simulink library "ee_lib_for_power_systems"  (R2025b)
settings: Solver=VariableStepAuto  StartTime=0.0  StopTime=10.0  RelTol=1e-3
undefined symbols (not defined in the file or in its folder): Alt_PolePair_Count, C3m, DiscIntNeverNeededParam, Enum1to12, L3m, Total_SM_Inertia, Ts
libraries: fl_lib x291, nesl_utility x219, ee_lib x139, custom_lib x12, $bdroot x10, ee_sl_lib x3, eeTransforms x2, eeGeneralControl x1, simulink x1
(part 9 of 11)

== system ee_lib_for_power_systems/Sense and Measure/voltage sensor ==
  PS-Simulink Converter1 : nesl_utility/PS-Simulink Converter  PhysicalDomain=network_engine_domain  SubClassName=ps_output  LeftPortType=input  RightPortType=output  PseudoPeriodic=off  Frequencies=[]  VectorFormat=inherit  Unit=inherit  AffineConversion=off
  Voltage Sensor : fl_lib/Electrical/Electrical Sensors/Voltage Sensor
  + : PMIOPort  Side=Left
  - : PMIOPort  Port=2  Side=Right
  v : Outport
  wires:
  PS-Simulink Converter1/1 -> v/1
  connections:
  Voltage Sensor/RConn2 -- -/RConn1
  PS-Simulink Converter1/LConn1 -- Voltage Sensor/RConn1
  Voltage Sensor/LConn1 -- +/RConn1

== system ee_lib_for_power_systems/Sources/Controlled Current Source ==
  CCS : Inport
  Controlled Current Source : fl_lib/Electrical/Electrical Sources/Controlled Current Source
  Simulink-PS Converter1 : nesl_utility/Simulink-PS Converter  PhysicalDomain=network_engine_domain  SubClassName=ps_input  LeftPortType=input  RightPortType=output  PseudoPeriodic=off  Frequencies=[]  NoiseDistribution=none  NoiseParameters=[]  Unit=1  AffineConversion=off  FilteringAndDerivatives=zero  SimscapeFilterOrder=1  InputFilterTimeConstant=0.001  UdotUserProvided=0
  + : PMIOPort  Side=Left
  - : PMIOPort  Port=2  Side=Right
  wires:
  CCS/1 -> Simulink-PS Converter1/1
  connections:
  Controlled Current Source/RConn2 -- -/RConn1
  Simulink-PS Converter1/RConn1 -- Controlled Current Source/RConn1
  Controlled Current Source/LConn1 -- +/RConn1

== system ee_lib_for_power_systems/Sources/Controlled Voltage Source ==
  CVS : Inport
  Controlled Voltage Source : fl_lib/Electrical/Electrical Sources/Controlled Voltage Source
  Simulink-PS Converter : nesl_utility/Simulink-PS Converter  PhysicalDomain=network_engine_domain  SubClassName=ps_input  LeftPortType=input  RightPortType=output  PseudoPeriodic=off  Frequencies=[]  NoiseDistribution=none  NoiseParameters=[]  Unit=1  AffineConversion=off  FilteringAndDerivatives=zero  SimscapeFilterOrder=1  InputFilterTimeConstant=0.001  UdotUserProvided=0
  + : PMIOPort  Side=Left
  - : PMIOPort  Port=2  Side=Right
  wires:
  CVS/1 -> Simulink-PS Converter/1
  connections:
  Simulink-PS Converter/RConn1 -- Controlled Voltage Source/RConn1
  Controlled Voltage Source/LConn1 -- +/RConn1
  Controlled Voltage Source/RConn2 -- -/RConn1

== system ee_lib_for_power_systems/Sources/Fuel Cell ==
  pfuel : Inport
  pair : Inport  Port=2
  qfuel : Inport  Port=3
  qair : Inport  Port=4
  Fuel Cell : ee_lib/Sources/Fuel Cell  model_fidelity=ee.enum.fuelcell.fidelity.detailed  Eoc=65 V  A=0.23 V  ri=0.05 Ohm  i0=80 A  i_lim=200 A  N_cell=65  number_unit=10  pH2_nom=1.5e5 Pa  pO2_nom=1.0e5 Pa  xH2=99  xO2=21  xW=1  dyn=ee.enum.include.no  Rd=0.005 Ohm  tau=10 s  Tnom=293.15 K  thermal_mass=30000 J/K  iFC_specify=off  iFC_priority=None  iFC=0 A  iFC_nominal_specify=off  iFC_nominal_value=1  iFC_nominal_unit=A  vFC_specify=off  vFC_priority=None  vFC=0 V  vFC_nominal_specify=off  vFC_nominal_value=1  vFC_nominal_unit=V  temperature_specify=off  temperature_priority=High  temperature=293 K  temperature_nominal_specify=off  temperature_nominal_value=1  temperature_nominal_unit=K
      settings: InternalSimscapePortConfiguration="{\"Bottom\":[],\"Left\":[{\"id\":\"H\",\"label\":\"H\",\"type\":\"foundation.thermal.thermal\"},{\"id\":\"pfuel\",\"label\":\"pfuel\",\"type\":\"input\"},{\"id\":\"pair\",\"label\":\"pair\",\"type\":\"input\"},{\"id\":\"qfuel\",\"label\":\"qfuel\",\"type\":\"input\"},{\"id\":\"qair\",\"label\":\"qair\",\"type\":\"input\"}],\"Right\":[{\"id\":\"p\",\"label\":\"+\",\"type\":\"foundation.electrical.electrical\"},{\"id\":\"n\",\"label\":\"-\",\"type\":\"foundation.electrical.electrical\"}],\"Top\":[]}"
  Simulink-PS Converter{,1..3} (4 alike) : nesl_utility/Simulink-PS Converter  PhysicalDomain=network_engine_domain  SubClassName=ps_input  LeftPortType=input  RightPortType=output  PseudoPeriodic=off  Frequencies=[]  NoiseDistribution=none  NoiseParameters=[]  Unit=1  AffineConversion=off  FilteringAndDerivatives=zero  SimscapeFilterOrder=1  InputFilterTimeConstant=0.001  UdotUserProvided=0
  Thermal Reference : fl_lib/Thermal/Thermal Elements/Thermal Reference
  + : PMIOPort  Side=Right
  - : PMIOPort  Port=2  Side=Right
  wires:
  pair/1 -> Simulink-PS Converter1/1
  qfuel/1 -> Simulink-PS Converter2/1
  pfuel/1 -> Simulink-PS Converter/1
  qair/1 -> Simulink-PS Converter3/1
  connections:
  Simulink-PS Converter/RConn1 -- Fuel Cell/LConn2
  Fuel Cell/RConn1 -- +/RConn1
  Simulink-PS Converter3/RConn1 -- Fuel Cell/LConn5
  Fuel Cell/LConn3 -- Simulink-PS Converter1/RConn1
  Simulink-PS Converter2/RConn1 -- Fuel Cell/LConn4
  Thermal Reference/LConn1 -- Fuel Cell/LConn1
  Fuel Cell/RConn2 -- -/RConn1

== system ee_lib_for_power_systems/Sources/Solar Array ==
  Ir : Inport
  Simulink-PS Converter : nesl_utility/Simulink-PS Converter  PhysicalDomain=network_engine_domain  SubClassName=ps_input  LeftPortType=input  RightPortType=output  PseudoPeriodic=off  Frequencies=[]  NoiseDistribution=none  NoiseParameters=[]  Unit=1  AffineConversion=off  FilteringAndDerivatives=zero  SimscapeFilterOrder=1  InputFilterTimeConstant=0.001  UdotUserProvided=0
  Solar Cell : ee_lib/Sources/Solar Cell  prm=ee.enum.sources.solar_cell_prm.datasheet  Isc=7.34 A  Voc=0.6 V  Is=1e-6 A  Is2=0 A  Iph=7.34 A  Ir0=1000 W/m^2  ec=1.5  N2=2  Rs=0 Ohm  Rp=inf Ohm  N_series=1  N_parallel=1  TIPH1=0 1/K  EG=1.11 eV  TXIS1=3  TXIS2=3  TRS1=0  TRP1=0  Tmeas=25 degC  TFIXED=25 degC  thermal_mass=100 J/K  initial_temperature=25 degC
  + : PMIOPort  Side=Right
  - : PMIOPort  Port=2  Side=Right
  wires:
  Ir/1 -> Simulink-PS Converter/1
  connections:
  Simulink-PS Converter/RConn1 -- Solar Cell/LConn1
  Solar Cell/LConn2 -- +/RConn1
  Solar Cell/RConn1 -- -/RConn1

== system ee_lib_for_power_systems/Transformations/Vectorized Park Transform ==
  abc : Inport
  theta : Inport  Port=2
  Constant : Constant  Value=-2*pi/3*ones(1,no_meas)  VectorParams1D=off  SampleTime=Ts
  Constant1 : Constant  Value=2*pi/3*ones(1,no_meas)  VectorParams1D=off  SampleTime=Ts
  Constant2 : Constant  Value=0.5*ones(1,no_meas)  VectorParams1D=off  SampleTime=Ts
  Constant3 : Constant  Value=0.5*ones(1,no_meas)  VectorParams1D=off  SampleTime=Ts
  Constant4 : Constant  Value=0.5*ones(1,no_meas)  VectorParams1D=off  SampleTime=Ts
  Gain : Gain  Gain=2/3
  Gain1 : Gain  Gain=2/3
  Gain2 : Gain  Gain=2/3
  Product : Product
  Product1 : Product
  Product2 : Product
  Sin : Trigonometry
  Sin1 : Trigonometry
  Sin2 : Trigonometry  Operator=cos
  Sin3 : Trigonometry  Operator=cos
  Sin6 : Trigonometry
  Sin7 : Trigonometry  Operator=cos
  Sum{1,5..7} (4 alike) : Sum  Inputs=++|
  Sum2 : Sum  Inputs=1  CollapseMode=Specified dimension  InputSameDT=on  SaturateOnIntegerOverflow=on  OutDataTypeStr=Inherit: Same as first input
  Sum3 : Sum  Inputs=1  CollapseMode=Specified dimension  InputSameDT=on  SaturateOnIntegerOverflow=on  OutDataTypeStr=Inherit: Same as first input
  Sum4 : Sum  Inputs=1  CollapseMode=Specified dimension  InputSameDT=on  SaturateOnIntegerOverflow=on  OutDataTypeStr=Inherit: Same as first input
  Vector Concatenate1 : Concatenate  NumInputs=3  Mode=Multidimensional array
  Vector Concatenate2 : Concatenate  NumInputs=3  Mode=Multidimensional array
  Vector Concatenate3 : Concatenate  NumInputs=3  Mode=Multidimensional array
  d : Outport  VectorParamsAs1DForOutWhenUnconnected=off
  q : Outport  Port=2  VectorParamsAs1DForOutWhenUnconnected=off
  0 : Outport  Port=3  VectorParamsAs1DForOutWhenUnconnected=off
  wires:
  theta/1 -> Sum7/1, Sin/1, Sum1/1, Sin2/1, Sum5/1, Sum6/1
  Sum7/1 -> Sin7/1
  Sum1/1 -> Sin3/1
  Constant1/1 -> Sum6/2, Sum7/2
  Constant/1 -> Sum5/2, Sum1/2
  Sum6/1 -> Sin6/1
  Sum5/1 -> Sin1/1
  abc/1 -> Product2/1, Product1/1, Product/1
  Sin/1 -> Vector Concatenate1/1
  Sin1/1 -> Vector Concatenate1/2
  Sin3/1 -> Vector Concatenate2/2
  Sin2/1 -> Vector Concatenate2/1
  Constant4/1 -> Vector Concatenate3/1
  Constant2/1 -> Vector Concatenate3/2
  Sin6/1 -> Vector Concatenate1/3
  Sin7/1 -> Vector Concatenate2/3
  Constant3/1 -> Vector Concatenate3/3
  Vector Concatenate3/1 -> Product2/2
  Vector Concatenate2/1 -> Product1/2
  Vector Concatenate1/1 -> Product/2
  Product/1 -> Sum2/1
  Product1/1 -> Sum3/1
  Product2/1 -> Sum4/1
  Sum3/1 -> Gain1/1
  Sum4/1 -> Gain2/1
  Sum2/1 -> Gain/1
  Gain2/1 -> 0/1
  Gain1/1 -> q/1
  Gain/1 -> d/1

== system ee_lib_for_power_systems/Transformations/Vectorized Inverse Park Transform ==
  dq0 : Inport
  theta : Inport  Port=2
  Constant : Constant  Value=-2*pi/3*ones(1,no_meas)  VectorParams1D=off  SampleTime=Ts
  Constant1 : Constant  Value=2*pi/3*ones(1,no_meas)  VectorParams1D=off  SampleTime=Ts
  Constant2 : Constant  Value=ones(1,no_meas)  VectorParams1D=off  SampleTime=Ts
  Constant3 : Constant  Value=ones(1,no_meas)  VectorParams1D=off  SampleTime=Ts
  Constant4 : Constant  Value=ones(1,no_meas)  VectorParams1D=off  SampleTime=Ts
  Product : Product
  Product1 : Product
  Product2 : Product
  Sin : Trigonometry
  Sin1 : Trigonometry  Operator=cos
  Sin2 : Trigonometry
  Sin3 : Trigonometry  Operator=cos
  Sin4 : Trigonometry
  Sin5 : Trigonometry  Operator=cos
  Sum : Sum  Inputs=++|
  Sum1 : Sum  Inputs=++|
  Sum2 : Sum  Inputs=1  CollapseMode=Specified dimension  InputSameDT=on  SaturateOnIntegerOverflow=on  OutDataTypeStr=Inherit: Same as first input
  Sum3 : Sum  Inputs=1  CollapseMode=Specified dimension  InputSameDT=on  SaturateOnIntegerOverflow=on  OutDataTypeStr=Inherit: Same as first input
  Sum4 : Sum  Inputs=1  CollapseMode=Specified dimension  InputSameDT=on  SaturateOnIntegerOverflow=on  OutDataTypeStr=Inherit: Same as first input
  Vector Concatenate1 : Concatenate  NumInputs=3  Mode=Multidimensional array
  Vector Concatenate2 : Concatenate  NumInputs=3  Mode=Multidimensional array
  Vector Concatenate3 : Concatenate  NumInputs=3  Mode=Multidimensional array
  a : Outport  VectorParamsAs1DForOutWhenUnconnected=off
  b : Outport  Port=2  VectorParamsAs1DForOutWhenUnconnected=off
  c : Outport  Port=3  VectorParamsAs1DForOutWhenUnconnected=off
  wires:
  Product2/1 -> Sum4/1
  Product1/1 -> Sum3/1
  Product/1 -> Sum2/1
  Vector Concatenate1/1 -> Product/2
  Vector Concatenate2/1 -> Product1/2
  Vector Concatenate3/1 -> Product2/2
  Constant3/1 -> Vector Concatenate3/3
  Constant4/1 -> Vector Concatenate2/3
  Constant2/1 -> Vector Concatenate1/3
  Sin5/1 -> Vector Concatenate3/2
  Sin4/1 -> Vector Concatenate3/1
  Sum1/1 -> Sin5/1, Sin4/1
  Constant1/1 -> Sum1/1
  Constant/1 -> Sum/1
  Sum/1 -> Sin2/1, Sin3/1
  Sin2/1 -> Vector Concatenate2/1
  Sin3/1 -> Vector Concatenate2/2
  Sin1/1 -> Vector Concatenate1/2
  Sin/1 -> Vector Concatenate1/1
  dq0/1 -> Product/1, Product1/1, Product2/1
  theta/1 -> Sum/2, Sin/1, Sum1/2, Sin1/1
  Sum2/1 -> a/1
  Sum3/1 -> b/1
  Sum4/1 -> c/1

== system ee_lib_for_power_systems/Utilities/Decoupling Bus ==
  V_in : Inport
  Controlled Voltage Source : fl_lib/Electrical/Electrical Sources/Controlled Voltage Source
  Controlled Voltage Source1 : fl_lib/Electrical/Electrical Sources/Controlled Voltage Source
  Controlled Voltage Source2 : fl_lib/Electrical/Electrical Sources/Controlled Voltage Source
  Demux : Demux  Outputs=3
  Electrical Reference2 : fl_lib/Electrical/Electrical Elements/Electrical Reference
  Mux1 : Mux  Inputs=3
  PS-Simulink Converter{,1,4..5,8..9} (6 alike) : nesl_utility/PS-Simulink Converter  PhysicalDomain=network_engine_domain  SubClassName=ps_output  LeftPortType=input  RightPortType=output  PseudoPeriodic=off  Frequencies=[]  VectorFormat=inherit  Unit=V*Hz*s  AffineConversion=off
  Phase Splitter : ee_lib/Connectors & References/Phase Splitter
  Resistor : fl_lib/Electrical/Electrical Elements/Resistor  R=Rcouple Ohm  i_specify=on  i_priority=Low  i=0 A  i_nominal_specify=off  i_nominal_value=1  i_nominal_unit=A  v_specify=on  v_priority=Low  v=0 V  v_nominal_specify=off  v_nominal_value=1  v_nominal_unit=V
  Resistor1 : fl_lib/Electrical/Electrical Elements/Resistor  R=Rcouple Ohm  i_specify=on  i_priority=Low  i=0 A  i_nominal_specify=off  i_nominal_value=1  i_nominal_unit=A  v_specify=on  v_priority=Low  v=0 V  v_nominal_specify=off  v_nominal_value=1  v_nominal_unit=V
  Resistor2 : fl_lib/Electrical/Electrical Elements/Resistor  R=Rcouple Ohm  i_specify=on  i_priority=Low  i=0 A  i_nominal_specify=off  i_nominal_value=1  i_nominal_unit=A  v_specify=on  v_priority=Low  v=0 V  v_nominal_specify=off  v_nominal_value=1  v_nominal_unit=V
  Simulink-PS Converter : nesl_utility/Simulink-PS Converter  PhysicalDomain=network_engine_domain  SubClassName=ps_input  LeftPortType=input  RightPortType=output  PseudoPeriodic=off  Frequencies=[]  NoiseDistribution=none  NoiseParameters=[]  Unit=1  AffineConversion=off  FilteringAndDerivatives=zero  SimscapeFilterOrder=1  InputFilterTimeConstant=0.001  UdotUserProvided=0
  Simulink-PS Converter1 : nesl_utility/Simulink-PS Converter  PhysicalDomain=network_engine_domain  SubClassName=ps_input  LeftPortType=input  RightPortType=output  PseudoPeriodic=off  Frequencies=[]  NoiseDistribution=none  NoiseParameters=[]  Unit=1  AffineConversion=off  FilteringAndDerivatives=zero  SimscapeFilterOrder=1  InputFilterTimeConstant=0.001  UdotUserProvided=0
  Simulink-PS Converter2 : nesl_utility/Simulink-PS Converter  PhysicalDomain=network_engine_domain  SubClassName=ps_input  LeftPortType=input  RightPortType=output  PseudoPeriodic=off  Frequencies=[]  NoiseDistribution=none  NoiseParameters=[]  Unit=1  AffineConversion=off  FilteringAndDerivatives=zero  SimscapeFilterOrder=1  InputFilterTimeConstant=0.001  UdotUserProvided=0
  Sum : Sum
  Sum1 : Sum
  Sum2 : Sum
  Voltage Sensor{,1..5} (6 alike) : fl_lib/Electrical/Electrical Sensors/Voltage Sensor
  ~ : PMIOPort  Side=Right
  V_out : Outport
  wires:
  PS-Simulink Converter5/1 -> Sum1/2
  PS-Simulink Converter1/1 -> Sum/2
  PS-Simulink Converter/1 -> Sum/1
  V_in/1 -> Demux/1
  Mux1/1 -> V_out/1
  Demux/3 -> Simulink-PS Converter1/1
  Demux/2 -> Simulink-PS Converter/1
  Demux/1 -> Simulink-PS Converter2/1
  Sum/1 -> Mux1/1
  PS-Simulink Converter4/1 -> Sum1/1
  Sum1/1 -> Mux1/2
  PS-Simulink Converter8/1 -> Sum2/1
  PS-Simulink Converter9/1 -> Sum2/2
  Sum2/1 -> Mux1/3
  connections:
  ~/RConn1 -- Phase Splitter/LConn1
  PS-Simulink Converter8/LConn1 -- Voltage Sensor5/RConn1
  PS-Simulink Converter9/LConn1 -- Voltage Sensor4/RConn1
  Voltage Sensor5/LConn1 -- Phase Splitter/RConn3 -- Resistor2/LConn1 -- Voltage Sensor4/LConn1
  Controlled Voltage Source2/LConn1 -- Voltage Sensor4/RConn2 -- Resistor2/RConn1
  Voltage Sensor1/RConn2 -- Voltage Sensor3/RConn2 -- Electrical Reference2/LConn1 -- Voltage Sensor5/RConn2 -- Controlled Voltage Source2/RConn2 -- Controlled Voltage Source1/RConn2 -- Controlled Voltage Source/RConn2
  PS-Simulink Converter5/LConn1 -- Voltage Sensor2/RConn1
  PS-Simulink Converter4/LConn1 -- Voltage Sensor3/RConn1
  Phase Splitter/RConn2 -- Voltage Sensor2/LConn1 -- Resistor1/LConn1 -- Voltage Sensor3/LConn1
  Controlled Voltage Source1/LConn1 -- Resistor1/RConn1 -- Voltage Sensor2/RConn2
  PS-Simulink Converter/LConn1 -- Voltage Sensor1/RConn1
  PS-Simulink Converter1/LConn1 -- Voltage Sensor/RConn1
  Voltage Sensor/LConn1 -- Voltage Sensor1/LConn1 -- Phase Splitter/RConn1 -- Resistor/LConn1
  Controlled Voltage Source/LConn1 -- Voltage Sensor/RConn2 -- Resistor/RConn1
  Simulink-PS Converter2/RConn1 -- Controlled Voltage Source/RConn1
  Simulink-PS Converter/RConn1 -- Controlled Voltage Source1/RConn1
  Simulink-PS Converter1/RConn1 -- Controlled Voltage Source2/RConn1

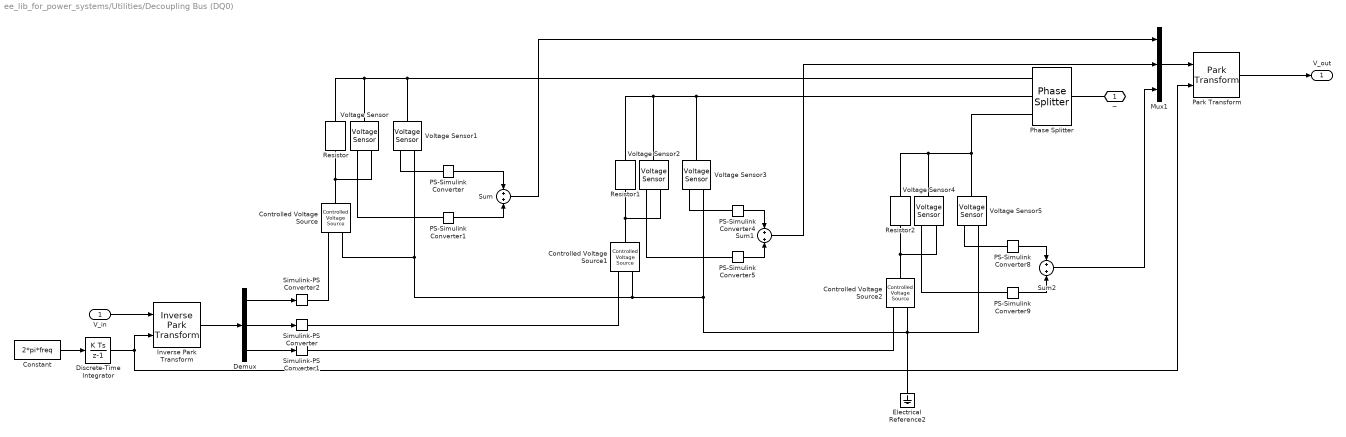
== system ee_lib_for_power_systems/Utilities/Decoupling Bus (DQ0) ==
  V_in : Inport
  Constant : Constant  Value=2*pi*freq  SampleTime=-1
  Controlled Voltage Source : fl_lib/Electrical/Electrical Sources/Controlled Voltage Source
  Controlled Voltage Source1 : fl_lib/Electrical/Electrical Sources/Controlled Voltage Source
  Controlled Voltage Source2 : fl_lib/Electrical/Electrical Sources/Controlled Voltage Source
  Demux : Demux  Outputs=3
  Discrete-Time Integrator : DiscreteIntegrator  SampleTime=-1  ICPrevOutput=DiscIntNeverNeededParam  ICPrevScaledInput=DiscIntNeverNeededParam
  Electrical Reference2 : fl_lib/Electrical/Electrical Elements/Electrical Reference
  Inverse Park Transform : eeTransforms/Inverse Park Transform  AxisAlignment=Q-axis  PowerInvariant=off
      settings: LibrarySourceBlock=|
      | ee_sl_lib/Mathematical
      | Transforms/Inverse
      | Park Transform
  Mux1 : Mux  Inputs=3
  PS-Simulink Converter{,1,4..5,8..9} (6 alike) : nesl_utility/PS-Simulink Converter  PhysicalDomain=network_engine_domain  SubClassName=ps_output  LeftPortType=input  RightPortType=output  PseudoPeriodic=off  Frequencies=[]  VectorFormat=inherit  Unit=V*Hz*s  AffineConversion=off
  Park Transform : eeTransforms/Park Transform  AxisAlignment=Q-axis  PowerInvariant=off
      settings: LibrarySourceBlock=|
      | ee_sl_lib/Mathematical
      | Transforms/Park Transform
  Phase Splitter : ee_lib/Connectors & References/Phase Splitter
  Resistor : fl_lib/Electrical/Electrical Elements/Resistor  R=Rcouple Ohm  i_specify=on  i_priority=Low  i=0 A  i_nominal_specify=off  i_nominal_value=1  i_nominal_unit=A  v_specify=on  v_priority=Low  v=0 V  v_nominal_specify=off  v_nominal_value=1  v_nominal_unit=V
  Resistor1 : fl_lib/Electrical/Electrical Elements/Resistor  R=Rcouple Ohm  i_specify=on  i_priority=Low  i=0 A  i_nominal_specify=off  i_nominal_value=1  i_nominal_unit=A  v_specify=on  v_priority=Low  v=0 V  v_nominal_specify=off  v_nominal_value=1  v_nominal_unit=V
  Resistor2 : fl_lib/Electrical/Electrical Elements/Resistor  R=Rcouple Ohm  i_specify=on  i_priority=Low  i=0 A  i_nominal_specify=off  i_nominal_value=1  i_nominal_unit=A  v_specify=on  v_priority=Low  v=0 V  v_nominal_specify=off  v_nominal_value=1  v_nominal_unit=V
  Simulink-PS Converter : nesl_utility/Simulink-PS Converter  PhysicalDomain=network_engine_domain  SubClassName=ps_input  LeftPortType=input  RightPortType=output  PseudoPeriodic=off  Frequencies=[]  NoiseDistribution=none  NoiseParameters=[]  Unit=1  AffineConversion=off  FilteringAndDerivatives=zero  SimscapeFilterOrder=1  InputFilterTimeConstant=0.001  UdotUserProvided=0
  Simulink-PS Converter1 : nesl_utility/Simulink-PS Converter  PhysicalDomain=network_engine_domain  SubClassName=ps_input  LeftPortType=input  RightPortType=output  PseudoPeriodic=off  Frequencies=[]  NoiseDistribution=none  NoiseParameters=[]  Unit=1  AffineConversion=off  FilteringAndDerivatives=zero  SimscapeFilterOrder=1  InputFilterTimeConstant=0.001  UdotUserProvided=0
  Simulink-PS Converter2 : nesl_utility/Simulink-PS Converter  PhysicalDomain=network_engine_domain  SubClassName=ps_input  LeftPortType=input  RightPortType=output  PseudoPeriodic=off  Frequencies=[]  NoiseDistribution=none  NoiseParameters=[]  Unit=1  AffineConversion=off  FilteringAndDerivatives=zero  SimscapeFilterOrder=1  InputFilterTimeConstant=0.001  UdotUserProvided=0
  Sum : Sum
  Sum1 : Sum
  Sum2 : Sum
  Voltage Sensor{,1..5} (6 alike) : fl_lib/Electrical/Electrical Sensors/Voltage Sensor
  ~ : PMIOPort  Side=Right
  V_out : Outport
  wires:
  Sum2/1 -> Mux1/3
  PS-Simulink Converter9/1 -> Sum2/2
  PS-Simulink Converter8/1 -> Sum2/1
  Sum1/1 -> Mux1/2
  PS-Simulink Converter4/1 -> Sum1/1
  Sum/1 -> Mux1/1
  Demux/1 -> Simulink-PS Converter2/1
  Demux/2 -> Simulink-PS Converter/1
  Demux/3 -> Simulink-PS Converter1/1
  PS-Simulink Converter/1 -> Sum/1
  PS-Simulink Converter1/1 -> Sum/2
  PS-Simulink Converter5/1 -> Sum1/2
  Inverse Park Transform/1 -> Demux/1
  V_in/1 -> Inverse Park Transform/1
  Discrete-Time Integrator/1 -> Inverse Park Transform/2, Park Transform/2
  Constant/1 -> Discrete-Time Integrator/1
  Mux1/1 -> Park Transform/1
  Park Transform/1 -> V_out/1
  connections:
  Simulink-PS Converter1/RConn1 -- Controlled Voltage Source2/RConn1
  Simulink-PS Converter/RConn1 -- Controlled Voltage Source1/RConn1
  Simulink-PS Converter2/RConn1 -- Controlled Voltage Source/RConn1
  Resistor/RConn1 -- Voltage Sensor/RConn2 -- Controlled Voltage Source/LConn1
  Resistor/LConn1 -- Phase Splitter/RConn1 -- Voltage Sensor1/LConn1 -- Voltage Sensor/LConn1
  PS-Simulink Converter1/LConn1 -- Voltage Sensor/RConn1
  PS-Simulink Converter/LConn1 -- Voltage Sensor1/RConn1
  Voltage Sensor2/RConn2 -- Resistor1/RConn1 -- Controlled Voltage Source1/LConn1
  Voltage Sensor3/LConn1 -- Voltage Sensor2/LConn1 -- Resistor1/LConn1 -- Phase Splitter/RConn2
  PS-Simulink Converter4/LConn1 -- Voltage Sensor3/RConn1
  PS-Simulink Converter5/LConn1 -- Voltage Sensor2/RConn1
  Controlled Voltage Source/RConn2 -- Controlled Voltage Source1/RConn2 -- Electrical Reference2/LConn1 -- Controlled Voltage Source2/RConn2 -- Voltage Sensor5/RConn2 -- Voltage Sensor3/RConn2 -- Voltage Sensor1/RConn2
  Resistor2/RConn1 -- Voltage Sensor4/RConn2 -- Controlled Voltage Source2/LConn1
  Voltage Sensor4/LConn1 -- Resistor2/LConn1 -- Phase Splitter/RConn3 -- Voltage Sensor5/LConn1
  PS-Simulink Converter9/LConn1 -- Voltage Sensor4/RConn1
  PS-Simulink Converter8/LConn1 -- Voltage Sensor5/RConn1
  ~/RConn1 -- Phase Splitter/LConn1

== system ee_lib_for_power_systems/Utilities/VF Replay PU (Three-Phase) ==
  V (pu) : Inport
  Freq (pu) : Inport  Port=2
  Constant : Constant  Value=[0 -1 1]*2*pi/3  SampleTime=Ts
  Controlled Voltage Source (Three-Phase) : ee_lib/Sources/Controlled Voltage Source (Three-Phase)  input_option=ee.enum.inputType.instantaneous  FRated=60 Hz
      settings: InternalSimscapePortConfiguration="{\"Bottom\":[],\"Left\":[{\"id\":\"S\",\"label\":\"S\",\"type\":\"input\"},{\"id\":\"n\",\"label\":\"n\",\"type\":\"foundation.electrical.electrical\"}],\"Right\":[{\"id\":\"N\",\"label\":\"~\",\"type\":\"foundation.electrical.three_phase\"}],\"Top\":[]}"
  Discrete-Time Integrator : DiscreteIntegrator  SampleTime=Ts  ICPrevOutput=DiscIntNeverNeededParam  ICPrevScaledInput=DiscIntNeverNeededParam
  Electrical Reference : fl_lib/Electrical/Electrical Elements/Electrical Reference
  Gain : Gain  Gain=2*pi*Fnom
  Gain1 : Gain  Gain=Vnom*sqrt(2/3)
  Product : Product
  Simulink-PS Converter : nesl_utility/Simulink-PS Converter  PhysicalDomain=network_engine_domain  SubClassName=ps_input  LeftPortType=input  RightPortType=output  PseudoPeriodic=off  Frequencies=[]  NoiseDistribution=none  NoiseParameters=[]  Unit=1  AffineConversion=off  FilteringAndDerivatives=zero  SimscapeFilterOrder=1  InputFilterTimeConstant=0.001  UdotUserProvided=0
  Sin : Trigonometry
  Sum : Sum  Inputs=|++
  ~ : PMIOPort  Side=Right
  wires:
  Gain1/1 -> Product/1
  V (pu)/1 -> Gain1/1
  Gain/1 -> Discrete-Time Integrator/1
  Discrete-Time Integrator/1 -> Sum/1
  Constant/1 -> Sum/2
  Sin/1 -> Product/2
  Sum/1 -> Sin/1
  Freq (pu)/1 -> Gain/1
  Product/1 -> Simulink-PS Converter/1
  connections:
  Controlled Voltage Source (Three-Phase)/RConn1 -- ~/RConn1
  Electrical Reference/LConn1 -- Controlled Voltage Source (Three-Phase)/LConn2
  Simulink-PS Converter/RConn1 -- Controlled Voltage Source (Three-Phase)/LConn1

== system ee_lib_for_power_systems/Utilities/VF Replay SI (Three-Phase) ==
  V (Vrms) : Inport
  Freq (Hz) : Inport  Port=2
  Constant : Constant  Value=[0 -1 1]*2*pi/3  SampleTime=Ts
  Controlled Voltage Source (Three-Phase) : ee_lib/Sources/Controlled Voltage Source (Three-Phase)  input_option=ee.enum.inputType.instantaneous  FRated=60 Hz
      settings: InternalSimscapePortConfiguration="{\"Bottom\":[],\"Left\":[{\"id\":\"S\",\"label\":\"S\",\"type\":\"input\"},{\"id\":\"n\",\"label\":\"n\",\"type\":\"foundation.electrical.electrical\"}],\"Right\":[{\"id\":\"N\",\"label\":\"~\",\"type\":\"foundation.electrical.three_phase\"}],\"Top\":[]}"
  Discrete-Time Integrator : DiscreteIntegrator  SampleTime=Ts  ICPrevOutput=DiscIntNeverNeededParam  ICPrevScaledInput=DiscIntNeverNeededParam
  Electrical Reference : fl_lib/Electrical/Electrical Elements/Electrical Reference
  Gain : Gain  Gain=2*pi
  Gain1 : Gain  Gain=sqrt(2/3)
  Product : Product
  Simulink-PS Converter : nesl_utility/Simulink-PS Converter  PhysicalDomain=network_engine_domain  SubClassName=ps_input  LeftPortType=input  RightPortType=output  PseudoPeriodic=off  Frequencies=[]  NoiseDistribution=none  NoiseParameters=[]  Unit=1  AffineConversion=off  FilteringAndDerivatives=zero  SimscapeFilterOrder=1  InputFilterTimeConstant=0.001  UdotUserProvided=0
  Sin : Trigonometry
  Sum : Sum  Inputs=|++
  ~ : PMIOPort  Side=Right
  wires:
  Product/1 -> Simulink-PS Converter/1
  Freq (Hz)/1 -> Gain/1
  Sum/1 -> Sin/1
  Sin/1 -> Product/2
  Constant/1 -> Sum/2
  Discrete-Time Integrator/1 -> Sum/1
  Gain/1 -> Discrete-Time Integrator/1
  V (Vrms)/1 -> Gain1/1
  Gain1/1 -> Product/1
  connections:
  Simulink-PS Converter/RConn1 -- Controlled Voltage Source (Three-Phase)/LConn1
  Electrical Reference/LConn1 -- Controlled Voltage Source (Three-Phase)/LConn2
  Controlled Voltage Source (Three-Phase)/RConn1 -- ~/RConn1

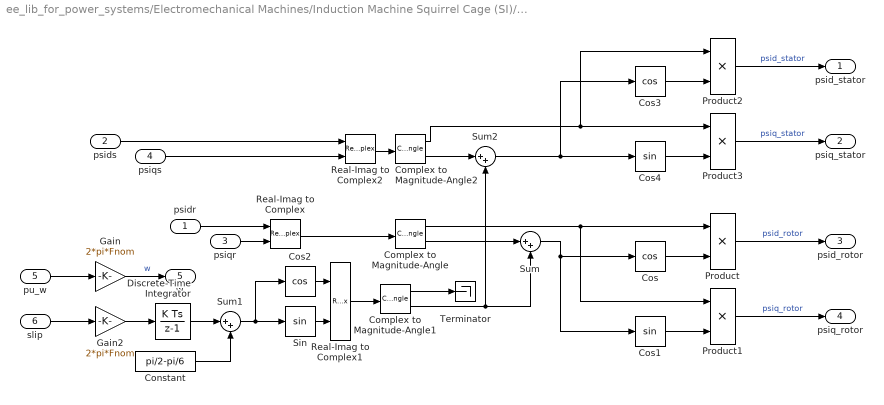
== system ee_lib_for_power_systems/Electromechanical Machines/Induction Machine Squirrel Cage (SI)/Subsystem ==
  psidr : Inport
  psids : Inport  Port=2
  psiqr : Inport  Port=3
  psiqs : Inport  Port=4
  pu_w : Inport  Port=5
  slip : Inport  Port=6
  Complex to Magnitude-Angle : ComplexToMagnitudeAngle
  Complex to Magnitude-Angle1 : ComplexToMagnitudeAngle
  Complex to Magnitude-Angle2 : ComplexToMagnitudeAngle
  Constant : Constant  Value=pi/2-pi/6  SampleTime=-1
  Cos : Trigonometry  Operator=cos
  Cos1 : Trigonometry
  Cos2 : Trigonometry  Operator=cos
  Cos3 : Trigonometry  Operator=cos
  Cos4 : Trigonometry
  Discrete-Time Integrator : DiscreteIntegrator  SampleTime=-1  ICPrevOutput=DiscIntNeverNeededParam  ICPrevScaledInput=DiscIntNeverNeededParam
  Gain : Gain  Gain=2*pi*Fnom
  Gain2 : Gain  Gain=2*pi*Fnom
  Product{,1..3} (4 alike) : Product
  Real-Imag to Complex : RealImagToComplex
  Real-Imag to Complex1 : RealImagToComplex
  Real-Imag to Complex2 : RealImagToComplex
  Sin : Trigonometry
  Sum : Sum  Inputs=|++
  Sum1 : Sum  Inputs=|++
  Sum2 : Sum  Inputs=|++
  Terminator : Terminator
  psid_stator : Outport
  psiq_stator : Outport  Port=2
  psid_rotor : Outport  Port=3
  psiq_rotor : Outport  Port=4
  w : Outport  Port=5
  wires:
  Cos/1 -> Product/2
  Gain2/1 -> Discrete-Time Integrator/1
  Sum2/1 -> Cos3/1, Cos4/1
  Constant/1 -> Sum1/2
  Sin/1 -> Real-Imag to Complex1/2
  Sum1/1 -> Cos2/1, Sin/1
  Complex to Magnitude-Angle2/1 -> Product2/1, Product3/1
  Complex to Magnitude-Angle/2 -> Sum/1
  Real-Imag to Complex1/1 -> Complex to Magnitude-Angle1/1
  Cos1/1 -> Product1/2
  Complex to Magnitude-Angle/1 -> Product1/1, Product/1
  psidr/1 -> Real-Imag to Complex/1
  Real-Imag to Complex/1 -> Complex to Magnitude-Angle/1
  Cos2/1 -> Real-Imag to Complex1/1
  Product/1 -> psid_rotor/1  "psid_rotor"
  Cos3/1 -> Product2/2
  Sum/1 -> Cos1/1, Cos/1
  Discrete-Time Integrator/1 -> Sum1/1
  Complex to Magnitude-Angle1/2 -> Sum2/2, Sum/2
  Cos4/1 -> Product3/2
  Product3/1 -> psiq_stator/1  "psiq_stator"
  psiqr/1 -> Real-Imag to Complex/2
  Real-Imag to Complex2/1 -> Complex to Magnitude-Angle2/1
  psiqs/1 -> Real-Imag to Complex2/2
  Complex to Magnitude-Angle2/2 -> Sum2/1
  pu_w/1 -> Gain/1
  Product2/1 -> psid_stator/1  "psid_stator"
  Product1/1 -> psiq_rotor/1  "psiq_rotor"
  psids/1 -> Real-Imag to Complex2/1
  Gain/1 -> w/1  "w"
  Complex to Magnitude-Angle1/1 -> Terminator/1
  slip/1 -> Gain2/1

== system ee_lib_for_power_systems/Electromechanical Machines/Round Rotor Standard (PU)/Meas ==
  Bus Creator : BusCreator  Inputs=4
  Mux : Mux  Inputs=2
  PS-Simulink Converter{,1..4} (5 alike) : nesl_utility/PS-Simulink Converter  PhysicalDomain=network_engine_domain  SubClassName=ps_output  LeftPortType=input  RightPortType=output  PseudoPeriodic=off  Frequencies=[]  VectorFormat=inherit  Unit=inherit  AffineConversion=off
  Sqrt : Sqrt
  Square : Math  Operator=square
  Sum : Sum  Inputs=+
  Synchronous Machine Measurement : ee_lib/Electromechanical/Synchronous/Synchronous Machine Measurement  output_idx=4
      settings: InternalSimscapePortConfiguration="{\"Bottom\":[],\"Left\":[{\"id\":\"pu_input\",\"label\":\"pu\",\"type\":\"input\"}],\"Right\":[{\"id\":\"pu_output\",\"label\":\"o\",\"type\":\"output\"}],\"Top\":[]}"
  Synchronous Machine Measurement1 : ee_lib/Electromechanical/Synchronous/Synchronous Machine Measurement  output_idx=5
      settings: InternalSimscapePortConfiguration="{\"Bottom\":[],\"Left\":[{\"id\":\"pu_input\",\"label\":\"pu\",\"type\":\"input\"}],\"Right\":[{\"id\":\"pu_output\",\"label\":\"o\",\"type\":\"output\"}],\"Top\":[]}"
  Synchronous Machine Measurement2 : ee_lib/Electromechanical/Synchronous/Synchronous Machine Measurement  output_idx=6
      settings: InternalSimscapePortConfiguration="{\"Bottom\":[],\"Left\":[{\"id\":\"pu_input\",\"label\":\"pu\",\"type\":\"input\"}],\"Right\":[{\"id\":\"pu_output\",\"label\":\"o\",\"type\":\"output\"}],\"Top\":[]}"
  Synchronous Machine Measurement3 : ee_lib/Electromechanical/Synchronous/Synchronous Machine Measurement  output_idx=12
      settings: InternalSimscapePortConfiguration="{\"Bottom\":[],\"Left\":[{\"id\":\"pu_input\",\"label\":\"pu\",\"type\":\"input\"}],\"Right\":[{\"id\":\"pu_output\",\"label\":\"o\",\"type\":\"output\"}],\"Top\":[]}"
  Synchronous Machine Measurement4 : ee_lib/Electromechanical/Synchronous/Synchronous Machine Measurement  output_idx=13
      settings: InternalSimscapePortConfiguration="{\"Bottom\":[],\"Left\":[{\"id\":\"pu_input\",\"label\":\"pu\",\"type\":\"input\"}],\"Right\":[{\"id\":\"pu_output\",\"label\":\"o\",\"type\":\"output\"}],\"Top\":[]}"
  m_PS : PMIOPort  Side=Left
  m : Outport
  wires:
  PS-Simulink Converter/1 -> Bus Creator/1  "w (pu)"
  Bus Creator/1 -> m/1
  PS-Simulink Converter1/1 -> Mux/1
  PS-Simulink Converter2/1 -> Mux/2
  Mux/1 -> Square/1
  Square/1 -> Sum/1
  Sum/1 -> Sqrt/1
  PS-Simulink Converter4/1 -> Bus Creator/4  "Q (pu)"
  Sqrt/1 -> Bus Creator/2  "Vt (pu)"
  PS-Simulink Converter3/1 -> Bus Creator/3  "P (pu)"
  connections:
  PS-Simulink Converter2/LConn1 -- Synchronous Machine Measurement2/RConn1
  PS-Simulink Converter/LConn1 -- Synchronous Machine Measurement/RConn1
  Synchronous Machine Measurement4/LConn1 -- Synchronous Machine Measurement3/LConn1 -- m_PS/RConn1 -- Synchronous Machine Measurement2/LConn1 -- Synchronous Machine Measurement1/LConn1 -- Synchronous Machine Measurement/LConn1
  PS-Simulink Converter4/LConn1 -- Synchronous Machine Measurement4/RConn1
  PS-Simulink Converter3/LConn1 -- Synchronous Machine Measurement3/RConn1
  PS-Simulink Converter1/LConn1 -- Synchronous Machine Measurement1/RConn1

== system ee_lib_for_power_systems/Electromechanical Machines/Salient Pole Fundamental (SI)/Meas ==
  Bus Creator : BusCreator  Inputs=7
  Gain : Gain  Gain=2*pi*Fnom/p
  Gain1 : Gain  Gain=Pnom/Vnom*sqrt(2/3)
  Gain2 : Gain  Gain=Vnom*sqrt(2/3)
  Gain3 : Gain  Gain=Vnom*sqrt(2/3)
  Gain4 : Gain  Gain=Pnom
  Gain5 : Gain  Gain=Pnom
  Mux : Mux  Inputs=2
  PS-Simulink Converter{,1..5} (6 alike) : nesl_utility/PS-Simulink Converter  PhysicalDomain=network_engine_domain  SubClassName=ps_output  LeftPortType=input  RightPortType=output  PseudoPeriodic=off  Frequencies=[]  VectorFormat=inherit  Unit=inherit  AffineConversion=off
  Sqrt : Sqrt
  Square : Math  Operator=square
  Sum : Sum  Inputs=+
  Synchronous Machine Measurement : ee_lib/Electromechanical/Synchronous/Synchronous Machine Measurement  output_idx=4
      settings: InternalSimscapePortConfiguration="{\"Bottom\":[],\"Left\":[{\"id\":\"pu_input\",\"label\":\"pu\",\"type\":\"input\"}],\"Right\":[{\"id\":\"pu_output\",\"label\":\"o\",\"type\":\"output\"}],\"Top\":[]}"
  Synchronous Machine Measurement1 : ee_lib/Electromechanical/Synchronous/Synchronous Machine Measurement  output_idx=5
      settings: InternalSimscapePortConfiguration="{\"Bottom\":[],\"Left\":[{\"id\":\"pu_input\",\"label\":\"pu\",\"type\":\"input\"}],\"Right\":[{\"id\":\"pu_output\",\"label\":\"o\",\"type\":\"output\"}],\"Top\":[]}"
  Synchronous Machine Measurement2 : ee_lib/Electromechanical/Synchronous/Synchronous Machine Measurement  output_idx=6
      settings: InternalSimscapePortConfiguration="{\"Bottom\":[],\"Left\":[{\"id\":\"pu_input\",\"label\":\"pu\",\"type\":\"input\"}],\"Right\":[{\"id\":\"pu_output\",\"label\":\"o\",\"type\":\"output\"}],\"Top\":[]}"
  Synchronous Machine Measurement3 : ee_lib/Electromechanical/Synchronous/Synchronous Machine Measurement  output_idx=12
      settings: InternalSimscapePortConfiguration="{\"Bottom\":[],\"Left\":[{\"id\":\"pu_input\",\"label\":\"pu\",\"type\":\"input\"}],\"Right\":[{\"id\":\"pu_output\",\"label\":\"o\",\"type\":\"output\"}],\"Top\":[]}"
  Synchronous Machine Measurement4 : ee_lib/Electromechanical/Synchronous/Synchronous Machine Measurement  output_idx=13
      settings: InternalSimscapePortConfiguration="{\"Bottom\":[],\"Left\":[{\"id\":\"pu_input\",\"label\":\"pu\",\"type\":\"input\"}],\"Right\":[{\"id\":\"pu_output\",\"label\":\"o\",\"type\":\"output\"}],\"Top\":[]}"
  Synchronous Machine Measurement5 : ee_lib/Electromechanical/Synchronous/Synchronous Machine Measurement  output_idx=2
      settings: InternalSimscapePortConfiguration="{\"Bottom\":[],\"Left\":[{\"id\":\"pu_input\",\"label\":\"pu\",\"type\":\"input\"}],\"Right\":[{\"id\":\"pu_output\",\"label\":\"o\",\"type\":\"output\"}],\"Top\":[]}"
  m_PS : PMIOPort  Side=Left
  m : Outport
  wires:
  Sqrt/1 -> Bus Creator/5  "Vt (V)"
  Sum/1 -> Sqrt/1
  Square/1 -> Sum/1
  Mux/1 -> Square/1
  PS-Simulink Converter2/1 -> Gain3/1
  PS-Simulink Converter1/1 -> Gain2/1
  Bus Creator/1 -> m/1
  PS-Simulink Converter/1 -> Gain/1  "w (pu)"
  PS-Simulink Converter5/1 -> Gain1/1  "Ifd (pu)"
  PS-Simulink Converter3/1 -> Gain4/1  "P (pu)"
  PS-Simulink Converter4/1 -> Gain5/1  "Q (pu)"
  Gain/1 -> Bus Creator/1  "w (rad/s)"
  Gain1/1 -> Bus Creator/2  "Ifd (A)"
  Gain2/1 -> Bus Creator/3, Mux/1  "Vd (V)"
  Gain3/1 -> Bus Creator/4, Mux/2  "Vq (V)"
  Gain4/1 -> Bus Creator/6  "P (W)"
  Gain5/1 -> Bus Creator/7  "Q (VAr)"
  connections:
  PS-Simulink Converter1/LConn1 -- Synchronous Machine Measurement1/RConn1
  PS-Simulink Converter3/LConn1 -- Synchronous Machine Measurement3/RConn1
  PS-Simulink Converter4/LConn1 -- Synchronous Machine Measurement4/RConn1
  Synchronous Machine Measurement5/LConn1 -- Synchronous Machine Measurement1/LConn1 -- Synchronous Machine Measurement2/LConn1 -- m_PS/RConn1 -- Synchronous Machine Measurement3/LConn1 -- Synchronous Machine Measurement4/LConn1 -- Synchronous Machine Measurement/LConn1
  PS-Simulink Converter/LConn1 -- Synchronous Machine Measurement/RConn1
  PS-Simulink Converter2/LConn1 -- Synchronous Machine Measurement2/RConn1
  PS-Simulink Converter5/LConn1 -- Synchronous Machine Measurement5/RConn1

== system ee_lib_for_power_systems/Electromechanical Machines/Salient Pole Standard (PU)/Meas ==
  Bus Creator : BusCreator  Inputs=4
  Mux : Mux  Inputs=2
  PS-Simulink Converter{,1..4} (5 alike) : nesl_utility/PS-Simulink Converter  PhysicalDomain=network_engine_domain  SubClassName=ps_output  LeftPortType=input  RightPortType=output  PseudoPeriodic=off  Frequencies=[]  VectorFormat=inherit  Unit=inherit  AffineConversion=off
  Sqrt : Sqrt
  Square : Math  Operator=square
  Sum : Sum  Inputs=+
  Synchronous Machine Measurement : ee_lib/Electromechanical/Synchronous/Synchronous Machine Measurement  output_idx=4
      settings: InternalSimscapePortConfiguration="{\"Bottom\":[],\"Left\":[{\"id\":\"pu_input\",\"label\":\"pu\",\"type\":\"input\"}],\"Right\":[{\"id\":\"pu_output\",\"label\":\"o\",\"type\":\"output\"}],\"Top\":[]}"
  Synchronous Machine Measurement1 : ee_lib/Electromechanical/Synchronous/Synchronous Machine Measurement  output_idx=5
      settings: InternalSimscapePortConfiguration="{\"Bottom\":[],\"Left\":[{\"id\":\"pu_input\",\"label\":\"pu\",\"type\":\"input\"}],\"Right\":[{\"id\":\"pu_output\",\"label\":\"o\",\"type\":\"output\"}],\"Top\":[]}"
  Synchronous Machine Measurement2 : ee_lib/Electromechanical/Synchronous/Synchronous Machine Measurement  output_idx=6
      settings: InternalSimscapePortConfiguration="{\"Bottom\":[],\"Left\":[{\"id\":\"pu_input\",\"label\":\"pu\",\"type\":\"input\"}],\"Right\":[{\"id\":\"pu_output\",\"label\":\"o\",\"type\":\"output\"}],\"Top\":[]}"
  Synchronous Machine Measurement3 : ee_lib/Electromechanical/Synchronous/Synchronous Machine Measurement  output_idx=12
      settings: InternalSimscapePortConfiguration="{\"Bottom\":[],\"Left\":[{\"id\":\"pu_input\",\"label\":\"pu\",\"type\":\"input\"}],\"Right\":[{\"id\":\"pu_output\",\"label\":\"o\",\"type\":\"output\"}],\"Top\":[]}"
  Synchronous Machine Measurement4 : ee_lib/Electromechanical/Synchronous/Synchronous Machine Measurement  output_idx=13
      settings: InternalSimscapePortConfiguration="{\"Bottom\":[],\"Left\":[{\"id\":\"pu_input\",\"label\":\"pu\",\"type\":\"input\"}],\"Right\":[{\"id\":\"pu_output\",\"label\":\"o\",\"type\":\"output\"}],\"Top\":[]}"
  m_PS : PMIOPort  Side=Left
  m : Outport
  wires:
  PS-Simulink Converter3/1 -> Bus Creator/3  "P (pu)"
  Sqrt/1 -> Bus Creator/2  "Vt (pu)"
  PS-Simulink Converter4/1 -> Bus Creator/4  "Q (pu)"
  Sum/1 -> Sqrt/1
  Square/1 -> Sum/1
  Mux/1 -> Square/1
  PS-Simulink Converter2/1 -> Mux/2
  PS-Simulink Converter1/1 -> Mux/1
  Bus Creator/1 -> m/1
  PS-Simulink Converter/1 -> Bus Creator/1  "w (pu)"
  connections:
  PS-Simulink Converter1/LConn1 -- Synchronous Machine Measurement1/RConn1
  PS-Simulink Converter3/LConn1 -- Synchronous Machine Measurement3/RConn1
  PS-Simulink Converter4/LConn1 -- Synchronous Machine Measurement4/RConn1
  Synchronous Machine Measurement/LConn1 -- Synchronous Machine Measurement1/LConn1 -- Synchronous Machine Measurement2/LConn1 -- m_PS/RConn1 -- Synchronous Machine Measurement3/LConn1 -- Synchronous Machine Measurement4/LConn1
  PS-Simulink Converter/LConn1 -- Synchronous Machine Measurement/RConn1
  PS-Simulink Converter2/LConn1 -- Synchronous Machine Measurement2/RConn1

== system ee_lib_for_power_systems/Electromechanical Machines/Salient Pole Standard (SI)/Meas ==
  Bus Creator : BusCreator  Inputs=7
  Mux : Mux  Inputs=2
  PS-Simulink Converter{,1..5} (6 alike) : nesl_utility/PS-Simulink Converter  PhysicalDomain=network_engine_domain  SubClassName=ps_output  LeftPortType=input  RightPortType=output  PseudoPeriodic=off  Frequencies=[]  VectorFormat=inherit  Unit=inherit  AffineConversion=off
  Sqrt : Sqrt
  Square : Math  Operator=square
  Sum : Sum  Inputs=+
  Synchronous Machine Measurement : ee_lib/Electromechanical/Synchronous/Synchronous Machine Measurement  output_idx=4
      settings: InternalSimscapePortConfiguration="{\"Bottom\":[],\"Left\":[{\"id\":\"pu_input\",\"label\":\"pu\",\"type\":\"input\"}],\"Right\":[{\"id\":\"pu_output\",\"label\":\"o\",\"type\":\"output\"}],\"Top\":[]}"
  Synchronous Machine Measurement1 : ee_lib/Electromechanical/Synchronous/Synchronous Machine Measurement  output_idx=5
      settings: InternalSimscapePortConfiguration="{\"Bottom\":[],\"Left\":[{\"id\":\"pu_input\",\"label\":\"pu\",\"type\":\"input\"}],\"Right\":[{\"id\":\"pu_output\",\"label\":\"o\",\"type\":\"output\"}],\"Top\":[]}"
  Synchronous Machine Measurement2 : ee_lib/Electromechanical/Synchronous/Synchronous Machine Measurement  output_idx=6
      settings: InternalSimscapePortConfiguration="{\"Bottom\":[],\"Left\":[{\"id\":\"pu_input\",\"label\":\"pu\",\"type\":\"input\"}],\"Right\":[{\"id\":\"pu_output\",\"label\":\"o\",\"type\":\"output\"}],\"Top\":[]}"
  Synchronous Machine Measurement3 : ee_lib/Electromechanical/Synchronous/Synchronous Machine Measurement  output_idx=12
      settings: InternalSimscapePortConfiguration="{\"Bottom\":[],\"Left\":[{\"id\":\"pu_input\",\"label\":\"pu\",\"type\":\"input\"}],\"Right\":[{\"id\":\"pu_output\",\"label\":\"o\",\"type\":\"output\"}],\"Top\":[]}"
  Synchronous Machine Measurement4 : ee_lib/Electromechanical/Synchronous/Synchronous Machine Measurement  output_idx=13
      settings: InternalSimscapePortConfiguration="{\"Bottom\":[],\"Left\":[{\"id\":\"pu_input\",\"label\":\"pu\",\"type\":\"input\"}],\"Right\":[{\"id\":\"pu_output\",\"label\":\"o\",\"type\":\"output\"}],\"Top\":[]}"
  Synchronous Machine Measurement5 : ee_lib/Electromechanical/Synchronous/Synchronous Machine Measurement  output_idx=2
      settings: InternalSimscapePortConfiguration="{\"Bottom\":[],\"Left\":[{\"id\":\"pu_input\",\"label\":\"pu\",\"type\":\"input\"}],\"Right\":[{\"id\":\"pu_output\",\"label\":\"o\",\"type\":\"output\"}],\"Top\":[]}"
  m_PS : PMIOPort  Side=Left
  m : Outport
  wires:
  PS-Simulink Converter4/1 -> Bus Creator/7  "Q (VA)"
  PS-Simulink Converter3/1 -> Bus Creator/6  "P (W)"
  Sqrt/1 -> Bus Creator/5  "Vt (V)"
  PS-Simulink Converter5/1 -> Bus Creator/2  "Ifd (A)"
  PS-Simulink Converter/1 -> Bus Creator/1  "w (rad/s)"
  Bus Creator/1 -> m/1
  PS-Simulink Converter1/1 -> Mux/1, Bus Creator/3  "Vd (V)"
  PS-Simulink Converter2/1 -> Mux/2, Bus Creator/4  "Vq (V)"
  Mux/1 -> Square/1
  Square/1 -> Sum/1
  Sum/1 -> Sqrt/1
  connections:
  PS-Simulink Converter5/LConn1 -- Synchronous Machine Measurement5/RConn1
  PS-Simulink Converter2/LConn1 -- Synchronous Machine Measurement2/RConn1
  PS-Simulink Converter/LConn1 -- Synchronous Machine Measurement/RConn1
  Synchronous Machine Measurement5/LConn1 -- Synchronous Machine Measurement/LConn1 -- Synchronous Machine Measurement4/LConn1 -- Synchronous Machine Measurement3/LConn1 -- m_PS/RConn1 -- Synchronous Machine Measurement2/LConn1 -- Synchronous Machine Measurement1/LConn1
  PS-Simulink Converter4/LConn1 -- Synchronous Machine Measurement4/RConn1
  PS-Simulink Converter3/LConn1 -- Synchronous Machine Measurement3/RConn1
  PS-Simulink Converter1/LConn1 -- Synchronous Machine Measurement1/RConn1

== system ee_lib_for_power_systems/Electromechanical Machines/Vectorized Simulink Synchronous Machine (PU)/Inverse Park Transform ==
  dq0 : Inport
  theta : Inport  Port=2
  Constant : Constant  Value=-2*pi/3*ones(1,no_meas)  VectorParams1D=off  SampleTime=Ts
  Constant1 : Constant  Value=2*pi/3*ones(1,no_meas)  VectorParams1D=off  SampleTime=Ts
  Constant2 : Constant  Value=ones(1,no_meas)  VectorParams1D=off  SampleTime=Ts
  Constant3 : Constant  Value=ones(1,no_meas)  VectorParams1D=off  SampleTime=Ts
  Constant4 : Constant  Value=ones(1,no_meas)  VectorParams1D=off  SampleTime=Ts
  Product : Product
  Product1 : Product
  Product2 : Product
  Sin : Trigonometry
  Sin1 : Trigonometry  Operator=cos
  Sin2 : Trigonometry
  Sin3 : Trigonometry  Operator=cos
  Sin4 : Trigonometry
  Sin5 : Trigonometry  Operator=cos
  Sum : Sum  Inputs=++|
  Sum1 : Sum  Inputs=++|
  Sum2 : Sum  Inputs=1  CollapseMode=Specified dimension  InputSameDT=on  SaturateOnIntegerOverflow=on  OutDataTypeStr=Inherit: Same as first input
  Sum3 : Sum  Inputs=1  CollapseMode=Specified dimension  InputSameDT=on  SaturateOnIntegerOverflow=on  OutDataTypeStr=Inherit: Same as first input
  Sum4 : Sum  Inputs=1  CollapseMode=Specified dimension  InputSameDT=on  SaturateOnIntegerOverflow=on  OutDataTypeStr=Inherit: Same as first input
  Vector Concatenate1 : Concatenate  NumInputs=3  Mode=Multidimensional array
  Vector Concatenate2 : Concatenate  NumInputs=3  Mode=Multidimensional array
  Vector Concatenate3 : Concatenate  NumInputs=3  Mode=Multidimensional array
  a : Outport  VectorParamsAs1DForOutWhenUnconnected=off
  b : Outport  Port=2  VectorParamsAs1DForOutWhenUnconnected=off
  c : Outport  Port=3  VectorParamsAs1DForOutWhenUnconnected=off
  wires:
  Product2/1 -> Sum4/1
  Product1/1 -> Sum3/1
  Product/1 -> Sum2/1
  Vector Concatenate1/1 -> Product/2
  Vector Concatenate2/1 -> Product1/2
  Vector Concatenate3/1 -> Product2/2
  Constant3/1 -> Vector Concatenate3/3
  Constant4/1 -> Vector Concatenate2/3
  Constant2/1 -> Vector Concatenate1/3
  Sin5/1 -> Vector Concatenate3/2
  Sin4/1 -> Vector Concatenate3/1
  Sum1/1 -> Sin5/1, Sin4/1
  Constant1/1 -> Sum1/1
  Constant/1 -> Sum/1
  Sum/1 -> Sin2/1, Sin3/1
  Sin2/1 -> Vector Concatenate2/1
  Sin3/1 -> Vector Concatenate2/2
  Sin1/1 -> Vector Concatenate1/2
  Sin/1 -> Vector Concatenate1/1
  dq0/1 -> Product/1, Product1/1, Product2/1
  theta/1 -> Sum/2, Sin/1, Sum1/2, Sin1/1
  Sum2/1 -> a/1
  Sum3/1 -> b/1
  Sum4/1 -> c/1

== system ee_lib_for_power_systems/Electromechanical Machines/Vectorized Simulink Synchronous Machine (PU)/PQ meas ==
  Vabc : Inport
  Iabc : Inport  Port=2
  Product1 : Product  InputSameDT=on  SaturateOnIntegerOverflow=on  OutDataTypeStr=Inherit: Same as first input
  Product3 : Product  InputSameDT=on  SaturateOnIntegerOverflow=on  OutDataTypeStr=Inherit: Same as first input
  Sum1 : Sum  Inputs=1  CollapseMode=Specified dimension  InputSameDT=on  SaturateOnIntegerOverflow=on  OutDataTypeStr=Inherit: Same as first input
  Sum2 : Sum  Inputs=1  CollapseMode=Specified dimension  InputSameDT=on  SaturateOnIntegerOverflow=on  OutDataTypeStr=Inherit: Same as first input
  Sum4 : Sum  Inputs=+-  InputSameDT=on  SaturateOnIntegerOverflow=on  OutDataTypeStr=Inherit: Same as first input
  pu->V : Gain  Gain=1/sqrt(3)  SaturateOnIntegerOverflow=on  OutDataTypeStr=Inherit: Same as input  ParamDataTypeStr=Inherit: Same as input
  pu->V1 : Gain  Gain=[0 1 0;0 0 1;1 0 0]  Multiplication=Matrix(K*u)  SaturateOnIntegerOverflow=on  OutDataTypeStr=Inherit: Same as input  ParamDataTypeStr=Inherit: Same as input
  pu->V2 : Gain  Gain=[0 1 0;0 0 1;1 0 0]  Multiplication=Matrix(K*u)  SaturateOnIntegerOverflow=on  OutDataTypeStr=Inherit: Same as input  ParamDataTypeStr=Inherit: Same as input
  Q : Outport  VectorParamsAs1DForOutWhenUnconnected=off
  P : Outport  Port=2  VectorParamsAs1DForOutWhenUnconnected=off
  wires:
  pu->V/1 -> Q/1
  Sum1/1 -> pu->V/1
  Product3/1 -> Sum1/1
  Product1/1 -> Sum2/1
  Vabc/1 -> Sum4/1, pu->V1/1, Product1/1  "VV1"
  Iabc/1 -> Product3/2, Product1/2
  Sum2/1 -> P/1
  pu->V1/1 -> Sum4/2
  Sum4/1 -> pu->V2/1  "VV2"
  pu->V2/1 -> Product3/1

== system ee_lib_for_power_systems/Electromechanical Machines/Vectorized Simulink Synchronous Machine (PU)/Stator side phase voltages --> qd voltages ==
  vABC : Inport
  theta : Inport  Port=2
  -> pu : Gain  Gain=[1./Vbase;1./Vbase;1./Vbase]
  Park Transform : Subsystem  mask{no_meas=no_gens}
      mask prompts: no_meas "Number of measurements"
      settings: LibrarySourceBlock=|
      | ee_sl_lib/Mathematical
      | Transforms/Inverse
      | Park Transform
  vqTer : Outport
  vdTer : Outport  Port=2
  wires:
  theta/1 -> Park Transform/2(theta)
  Park Transform/2(d) -> vdTer/1
  Park Transform/1(q) -> vqTer/1
  vABC/1 -> -> pu/1
  -> pu/1 -> Park Transform/1(abc)

== system ee_lib_for_power_systems/Network Components/3-Phase Branch Positive Sequence PU/Tx ==
  Electrical Reference : fl_lib/Electrical/Electrical Elements/Electrical Reference
  Electrical Reference1 : fl_lib/Electrical/Electrical Elements/Electrical Reference
  Phase Splitter : ee_lib/Connectors & References/Phase Splitter
  Phase Splitter1 : ee_lib/Connectors & References/Phase Splitter
  TtxA : fl_lib/Electrical/Electrical Elements/Ideal Transformer  n=wr  i1_specify=on  i1_priority=Low  i1=0 A  i1_nominal_specify=off  i1_nominal_value=1  i1_nominal_unit=A  v1_specify=on  v1_priority=Low  v1=0 V  v1_nominal_specify=off  v1_nominal_value=1  v1_nominal_unit=V  i2_specify=on  i2_priority=Low  i2=0 A  i2_nominal_specify=off  i2_nominal_value=1  i2_nominal_unit=A  v2_specify=on  v2_priority=Low  v2=0 V  v2_nominal_specify=off  v2_nominal_value=1  v2_nominal_unit=V
  TtxB : fl_lib/Electrical/Electrical Elements/Ideal Transformer  n=wr  i1_specify=on  i1_priority=Low  i1=0 A  i1_nominal_specify=off  i1_nominal_value=1  i1_nominal_unit=A  v1_specify=on  v1_priority=Low  v1=0 V  v1_nominal_specify=off  v1_nominal_value=1  v1_nominal_unit=V  i2_specify=on  i2_priority=Low  i2=0 A  i2_nominal_specify=off  i2_nominal_value=1  i2_nominal_unit=A  v2_specify=on  v2_priority=Low  v2=0 V  v2_nominal_specify=off  v2_nominal_value=1  v2_nominal_unit=V
  TtxC : fl_lib/Electrical/Electrical Elements/Ideal Transformer  n=wr  i1_specify=on  i1_priority=Low  i1=0 A  i1_nominal_specify=off  i1_nominal_value=1  i1_nominal_unit=A  v1_specify=on  v1_priority=Low  v1=0 V  v1_nominal_specify=off  v1_nominal_value=1  v1_nominal_unit=V  i2_specify=on  i2_priority=Low  i2=0 A  i2_nominal_specify=off  i2_nominal_value=1  i2_nominal_unit=A  v2_specify=on  v2_priority=Low  v2=0 V  v2_nominal_specify=off  v2_nominal_value=1  v2_nominal_unit=V
  ~1 : PMIOPort  Side=Left
  ~2 : PMIOPort  Port=2  Side=Right
  connections:
  TtxA/LConn2 -- TtxB/LConn2 -- Electrical Reference/LConn1 -- TtxC/LConn2
  Phase Splitter/RConn3 -- TtxC/LConn1
  TtxA/RConn2 -- Electrical Reference1/LConn1 -- TtxC/RConn2 -- TtxB/RConn2
  Phase Splitter1/RConn3 -- TtxC/RConn1
  Phase Splitter1/LConn1 -- ~2/RConn1
  Phase Splitter1/RConn2 -- TtxB/RConn1
  Phase Splitter/RConn2 -- TtxB/LConn1
  Phase Splitter/RConn1 -- TtxA/LConn1
  Phase Splitter1/RConn1 -- TtxA/RConn1
  Phase Splitter/LConn1 -- ~1/RConn1
